simulink model "AuxClimaCtlMgr"  (R2022b)
settings: Solver=FixedStepDiscrete  StartTime=0.0  StopTime=10.0  FixedStep=0.2  RelTol=1e-3
(part 1 of 2)
settings Model: Creator=blue.ge  LastModifiedBy=blue.ge
settings DataIOCC: SaveFormat=StructureWithTime
settings OptimizationCC: DefaultParameterBehavior=Inlined  EnhancedBackFolding=on  PassReuseOutputArgsAs=Individual arguments  ZeroExternalMemoryAtStartup=off  ZeroInternalMemoryAtStartup=off  OptimizeBlockOrder=speed  DifferentSizesBufferReuse=on  OptimizationCustomize=off  EfficientTunableParamExpr=on
settings DebuggingCC: ParameterTunabilityLossMsg=error
settings ModelReferenceCC: ModelReferenceNumInstancesAllowed=Single
settings SFSimCC: MATLABDynamicMemAlloc=off
settings RTWCC: Description=AUTOSAR  SystemTargetFile=autosar.tlc  GenCodeOnly=on  TemplateMakefile=ert_default_tmf
settings CodeAppCC: ForceParamTrailComments=on  IgnoreCustomStorageClasses=off  OperatorAnnotations=on  SimulinkDataObjDesc=on  SFDataObjDesc=on  InsertBlockDesc=on
settings STFCustomTargetCC: TargetLangStandard=C99 (ISO)  MultiwordLength=256  ParMdlRefBuildCompliant=on  CompOptLevelCompliant=on  IncludeMdlTerminateFcn=off  CombineOutputUpdateFcns=on  SuppressErrorStatus=on  ERTCustomFileBanners=on  SupportNonFinite=off  SupportComplex=off  SupportContinuousTime=off  SupportNonInlinedSFcns=off  RemoveResetFunc=on  MATLABClassNameForMDSCustomization=Simulink.SoftwareTarget.ERTCustomization  AutosarCompliant=on  UseToolchainInfoCompliant=on  LookupTableObjectStructAxisOrder=2,1,3,4,...  LUTObjectStructOrderEvenSpacing=Size,Table,Breakpoints  SystemTargetFile=autosar.tlc
settings ERTTargetCC: TargetLangStandard=C99 (ISO)  ConcurrentExecutionCompliant=off  IncludeMdlTerminateFcn=off  SuppressErrorStatus=on  SupportNonFinite=off  SupportComplex=off  ModelStepFunctionPrototypeControlCompliant=off  CPPClassGenCompliant=off  AutosarCompliant=on  LookupTableObjectStructAxisOrder=2,1,3,4,...  LUTObjectStructOrderEvenSpacing=Size,Table,Breakpoints  InlinedParameterPlacement=NonHierarchical  ERTCustomFileTemplate=example_file_process.tlc

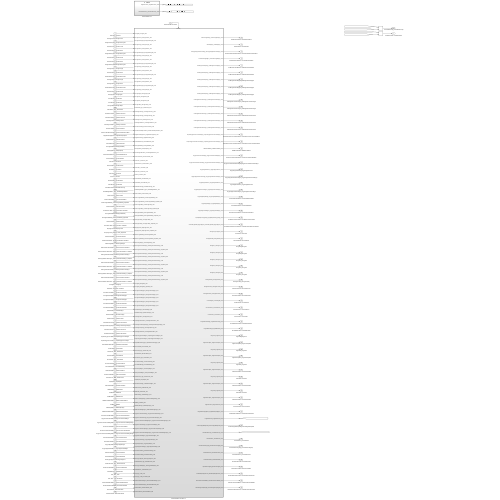
[thumbnail: Simulink's stored preview of the top-level diagram]
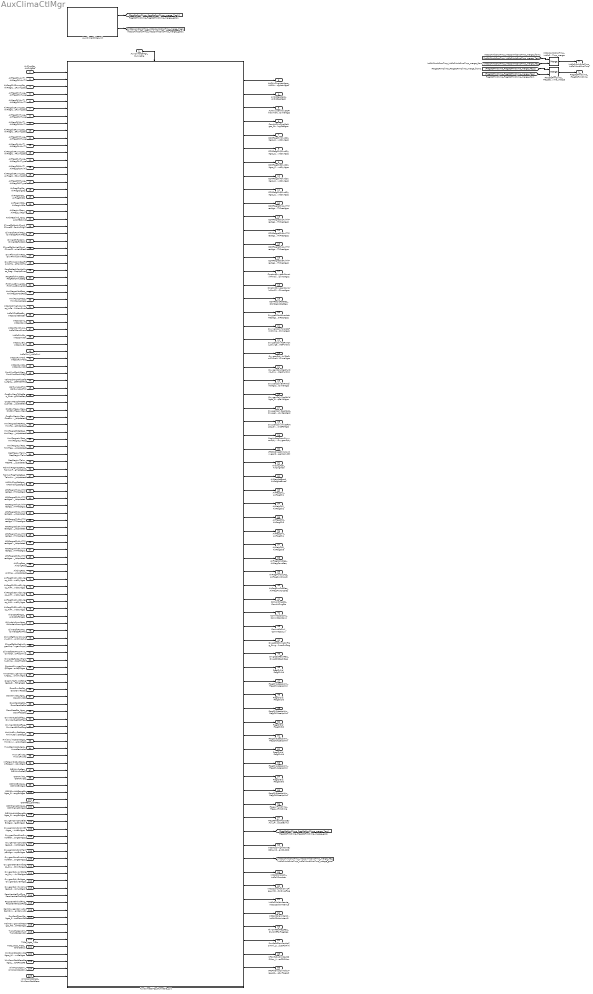
== system AuxClimaCtlMgr ==
  AuxClimaCtlMgr_Runnable : Inport  SampleTime=0.2  OutputFunctionCall=on
  AirClngSts_AirClngSts : Inport  Port=2  OutDataTypeStr=Enum: AirIonSts  PortDimensions=1
  AirFragCh1AvlTi_AirFragCh1AvlTi : Inport  Port=3  OutMin=0  OutMax=360  OutDataTypeStr=AfuTime  PortDimensions=1
  AirFragCh1RunngSts_AirFragCh1RunngSts : Inport  Port=4  OutDataTypeStr=Enum: OnOff1  PortDimensions=1
  AirFragCh1Type_AirFragCh1Type : Inport  Port=5  OutDataTypeStr=UInt8  PortDimensions=1
  AirFragCh2AvlTi_AirFragCh2AvlTi : Inport  Port=6  OutMin=0  OutMax=360  OutDataTypeStr=AfuTime  PortDimensions=1
  AirFragCh2RunngSts_AirFragCh2RunngSts : Inport  Port=7  OutDataTypeStr=Enum: OnOff1  PortDimensions=1
  AirFragCh2Type_AirFragCh2Type : Inport  Port=8  OutDataTypeStr=UInt8  PortDimensions=1
  AirFragCh3AvlTi_AirFragCh3AvlTi : Inport  Port=9  OutMin=0  OutMax=360  OutDataTypeStr=AfuTime  PortDimensions=1
  AirFragCh3RunngSts_AirFragCh3RunngSts : Inport  Port=10  OutDataTypeStr=Enum: OnOff1  PortDimensions=1
  AirFragCh3Type_AirFragCh3Type : Inport  Port=11  OutDataTypeStr=UInt8  PortDimensions=1
  AirFragCh4AvlTi_AirFragCh4AvlTi : Inport  Port=12  OutMin=0  OutMax=360  OutDataTypeStr=AfuTime  PortDimensions=1
  AirFragCh4RunngSts_AirFragCh4RunngSts : Inport  Port=13  OutDataTypeStr=Enum: OnOff1  PortDimensions=1
  AirFragCh4Type_AirFragCh4Type : Inport  Port=14  OutDataTypeStr=UInt8  PortDimensions=1
  AirFragCh5AvlTi_AirFragCh5AvlTi : Inport  Port=15  OutMin=0  OutMax=360  OutDataTypeStr=AfuTime  PortDimensions=1
  AirFragCh5RunngSts_AirFragCh5RunngSts : Inport  Port=16  OutDataTypeStr=Enum: OnOff1  PortDimensions=1
  AirFragCh5Type_AirFragCh5Type : Inport  Port=17  OutDataTypeStr=UInt8  PortDimensions=1
  AirFragChgSts_AirFragChgSts : Inport  Port=18  OutDataTypeStr=Enum: ChagSts  PortDimensions=1
  AirFragErrSts_AirFragErrSts : Inport  Port=19  OutDataTypeStr=Boolean  PortDimensions=1
  AirFragInitSts_AirFragInitSts : Inport  Port=20  OutDataTypeStr=Enum: InitSts  PortDimensions=1
  AirFragLvlRspn_AirFragLvlRspn : Inport  Port=21  OutDataTypeStr=Enum: RatUse  PortDimensions=1
  AmbTEstimd_Ngcc_AmbTEstimd : Inport  Port=22  OutDataTypeStr=Bus: AmbTEstimd  PortDimensions=1  BusOutputAsStruct=on
  ClimaCfgForAirClng1_ClimaCfgForAirClng1 : Inport  Port=23  OutDataTypeStr=Boolean  PortDimensions=1
  ClimaCfgForAirFrag_ClimaCfgForAirFrag : Inport  Port=24  OutDataTypeStr=Boolean  PortDimensions=1
  ClimaCfgForCds1_ClimaCfgForCds1 : Inport  Port=25  OutDataTypeStr=Boolean  PortDimensions=1
  ClimaCfgForVehElec1_ClimaCfgForVehElec1 : Inport  Port=26  OutDataTypeStr=Boolean  PortDimensions=1
  DrvrPfmncAlrmReq_DrvrPfmncAlrmReq : Inport  Port=27  OutDataTypeStr=Enum: DrvrPfmncWarnReq  PortDimensions=1
  DrvrPfmncAlrmReqFromDHU_DrvrPfmncAlrmReqFromDHU : Inport  Port=28  OutDataTypeStr=Enum: DrvrPfmncWarnReq  PortDimensions=1
  FragRefreshPopUpSucs_FragRefreshPopUpSucs : Inport  Port=29  OutDataTypeStr=Boolean  PortDimensions=1
  FragRefrshAutSetg_FragRefrshAutSetg : Inport  Port=30  OutDataTypeStr=Enum: OnOff1  PortDimensions=1
  FrntHvacBlowerSts_FrntHvacBlowerSts : Inport  Port=31  OutDataTypeStr=Enum: HvacFanSts  PortDimensions=1
  HmiFragraModReq_HmiFragraModReq : Inport  Port=32  OutDataTypeStr=Enum: HmiFragraModReq  PortDimensions=1
  HmiPopUpResp_HmiPopUpResp : Inport  Port=33  OutDataTypeStr=Enum: BookChargeSetResponse  PortDimensions=1
  InCarCo2HighWarnSucs_InCarCo2HighWarnSucs : Inport  Port=34  OutDataTypeStr=Boolean  PortDimensions=1
  IntCo2CircElecErr_IntCo2CircElecErr : Inport  Port=35  OutDataTypeStr=Enum: PmSnsrErrSts  PortDimensions=1
  IntCo2Conc_IntCo2Conc : Inport  Port=36  OutDataTypeStr=Co2Conc  PortDimensions=1
  IntCo2fromExsrc_IntCo2fromExsrc : Inport  Port=37  OutDataTypeStr=Enum: OnOff1  PortDimensions=1
  IntCo2HvErr_IntCo2HvErr : Inport  Port=38  OutDataTypeStr=Enum: Err1  PortDimensions=1
  IntCo2LvErr_IntCo2LvErr : Inport  Port=39  OutDataTypeStr=Enum: Err1  PortDimensions=1
  IntCo2Lvl_IntCo2Lvl : Inport  Port=40  OutDataTypeStr=Enum: Co2Lvl  PortDimensions=1
  IntCo2RunMod_IntCo2RunMod : Inport  Port=41  OutDataTypeStr=Enum: CdsRunngMod  PortDimensions=1
  IntCo2RunSts_IntCo2RunSts : Inport  Port=42  OutDataTypeStr=Enum: PmSnsrSts  PortDimensions=1
  PwrAllwdForAirFrag_PwrAllwdForAirFrag : Inport  Port=43  OutDataTypeStr=Pwr33  PortDimensions=1
  VehModMngtGlbSafe1_Ngcc_VehModMngtGlbSafe1 : Inport  Port=44  OutDataTypeStr=Bus: VehModMngtGlbSafe1  PortDimensions=1  BusOutputAsStruct=on
  VehSunLoadTot_VehSunLoadTot : Inport  Port=45  OutDataTypeStr=SunLoad30  PortDimensions=1
  CnctSrvFragChRatReq_CnctSrvFragChRatReq : Inport  Port=46  OutDataTypeStr=Bus: HmiFragraTypRatReq  PortDimensions=1  BusOutputAsStruct=on
  CnctSrvFragChRatReq_CnctSrvFragChRatReq_IsUpdated : Inport  Port=47  OutDataTypeStr=boolean  PortDimensions=1
  CnctSrvFragLvlReq_CnctSrvFragLvlReq : Inport  Port=48  OutDataTypeStr=Enum: HmiFragraLvlReq  PortDimensions=1
  CnctSrvFragLvlReq_CnctSrvFragLvlReq_IsUpdated : Inport  Port=49  OutDataTypeStr=boolean  PortDimensions=1
  HmiFragraChRatReq_HmiFragraChRatReq : Inport  Port=50  OutDataTypeStr=Bus: HmiFragraTypRatReq  PortDimensions=1  BusOutputAsStruct=on
  HmiFragraChRatReq_HmiFragraChRatReq_IsUpdated : Inport  Port=51  OutDataTypeStr=boolean  PortDimensions=1
  HmiFragraLvlReq_HmiFragraLvlReq : Inport  Port=52  OutDataTypeStr=Enum: HmiFragraLvlReq  PortDimensions=1
  HmiFragraLvlReq_HmiFragraLvlReq_IsUpdated : Inport  Port=53  OutDataTypeStr=boolean  PortDimensions=1
  ReqFragLvlTelm_ReqFragLvlTelm : Inport  Port=54  OutDataTypeStr=Enum: ReqFragLvlTelm  PortDimensions=1
  ReqFragLvlTelm_ReqFragLvlTelm_IsUpdated : Inport  Port=55  OutDataTypeStr=boolean  PortDimensions=1
  TelmAirFragTasteReq_TelmAirFragTasteReq : Inport  Port=56  OutDataTypeStr=Enum: TelmAirFragChReq  PortDimensions=1
  TelmAirFragTasteReq_TelmAirFragTasteReq_IsUpdated : Inport  Port=57  OutDataTypeStr=boolean  PortDimensions=1
  AACAirClngStsNgcc_AACAirClngStsNgcc : Inport  Port=58  OutDataTypeStr=Enum: AACAirClngSts  PortDimensions=1
  AFUFragraCh1AvlTiPrecNgcc_AFUFragraCh1AvlTiPrecNgcc : Inport  Port=59  OutMin=0  OutMax=100  OutDataTypeStr=Perc9  PortDimensions=1
  AFUFragraCh1AvlTiPrecNgcc_AFUFragraCh1AvlTiPrecNgcc_IsUpdated : Inport  Port=60  OutDataTypeStr=boolean  PortDimensions=1
  AFUFragraCh2AvlTiPrecNgcc_AFUFragraCh2AvlTiPrecNgcc : Inport  Port=61  OutMin=0  OutMax=100  OutDataTypeStr=Perc9  PortDimensions=1
  AFUFragraCh2AvlTiPrecNgcc_AFUFragraCh2AvlTiPrecNgcc_IsUpdated : Inport  Port=62  OutDataTypeStr=boolean  PortDimensions=1
  AFUFragraCh3AvlTiPrecNgcc_AFUFragraCh3AvlTiPrecNgcc : Inport  Port=63  OutMin=0  OutMax=100  OutDataTypeStr=Perc9  PortDimensions=1
  AFUFragraCh3AvlTiPrecNgcc_AFUFragraCh3AvlTiPrecNgcc_IsUpdated : Inport  Port=64  OutDataTypeStr=boolean  PortDimensions=1
  AFUFragraCh4AvlTiPrecNgcc_AFUFragraCh4AvlTiPrecNgcc : Inport  Port=65  OutMin=0  OutMax=100  OutDataTypeStr=Perc9  PortDimensions=1
  AFUFragraCh4AvlTiPrecNgcc_AFUFragraCh4AvlTiPrecNgcc_IsUpdated : Inport  Port=66  OutDataTypeStr=boolean  PortDimensions=1
  AFUFragraCh5AvlTiPrecNgcc_AFUFragraCh5AvlTiPrecNgcc : Inport  Port=67  OutMin=0  OutMax=100  OutDataTypeStr=Perc9  PortDimensions=1
  AFUFragraCh5AvlTiPrecNgcc_AFUFragraCh5AvlTiPrecNgcc_IsUpdated : Inport  Port=68  OutDataTypeStr=boolean  PortDimensions=1
  AirClngReq_AirClngReq : Inport  Port=69  OutDataTypeStr=Enum: OnOffNoReq  PortDimensions=1
  AirClngReq_AirClngReq_IsUpdated : Inport  Port=70  OutDataTypeStr=boolean  PortDimensions=1
  AirFragCh1OvrdDyNgcc_AirFragCh1OvrdDyNgcc : Inport  Port=71  OutDataTypeStr=Bus: DateTi30  PortDimensions=1  BusOutputAsStruct=on
  AirFragCh2OvrdDyNgcc_AirFragCh2OvrdDyNgcc : Inport  Port=72  OutDataTypeStr=Bus: DateTi30  PortDimensions=1  BusOutputAsStruct=on
  AirFragCh3OvrdDyNgcc_AirFragCh3OvrdDyNgcc : Inport  Port=73  OutDataTypeStr=Bus: DateTi30  PortDimensions=1  BusOutputAsStruct=on
  AirFragCh4OvrdDyNgcc_AirFragCh4OvrdDyNgcc : Inport  Port=74  OutDataTypeStr=Bus: DateTi30  PortDimensions=1  BusOutputAsStruct=on
  AirFragCh5OvrdDyNgcc_AirFragCh5OvrdDyNgcc : Inport  Port=75  OutDataTypeStr=Bus: DateTi30  PortDimensions=1  BusOutputAsStruct=on
  AirSrcStsFbNgcc_AirSrcStsFbNgcc : Inport  Port=76  OutMin=0  OutMax=3  OutDataTypeStr=UInt2  PortDimensions=1
  AltitudeInfromNgcc_AltitudeInfromNgcc : Inport  Port=77  OutDataTypeStr=UInt15  PortDimensions=1
  ClimaCfgForAAC_ClimaCfgForAAC : Inport  Port=78  OutDataTypeStr=Enum: CarCfgForAirClng  PortDimensions=1
  ClimaCfgForAirClnrAdv1_ClimaCfgForAirClnrAdv1 : Inport  Port=79  OutDataTypeStr=Boolean  PortDimensions=1
  ClimaCfgForCabinOxgenSupply_ClimaCfgForCabinOxgenSupply : Inport  Port=80  OutDataTypeStr=Enum: CabinOxgenSupply  PortDimensions=1
  ClimaCfgForPrpsnTyp_ClimaCfgForPrpsnTyp : Inport  Port=81  OutDataTypeStr=Enum: CarCfgForPrpsnTypGly  PortDimensions=1
  ClimaCfgForRoofHatch_ClimaCfgForRoofHatch : Inport  Port=82  OutDataTypeStr=Enum: CarCfgForRoofHatch  PortDimensions=1
  CmptmtOxygenConcQFNgcc_CmptmtOxygenConcQFNgcc : Inport  Port=83  OutDataTypeStr=Enum: Qf1  PortDimensions=1
  CmptmtOxygenConcVluNgcc_CmptmtOxygenConcVluNgcc : Inport  Port=84  OutMin=0  OutMax=100  OutDataTypeStr=Perc9  PortDimensions=1
  DispHvBattLvlOfChrgNgcc_DispHvBattLvlOfChrgNgcc : Inport  Port=85  OutMin=0  OutMax=102.30000000000001  OutDataTypeStr=EcoDrvInfo  PortDimensions=1
  DoorDrvrReSts_DoorDrvrReSts : Inport  Port=86  OutDataTypeStr=Enum: DoorSts2  PortDimensions=1
  DoorDrvrSts_Ngcc_DoorDrvrSts : Inport  Port=87  OutDataTypeStr=Enum: DoorSts2  PortDimensions=1
  DoorPassReSts_DoorPassReSts : Inport  Port=88  OutDataTypeStr=Enum: DoorSts2  PortDimensions=1
  DoorPassSts_Ngcc_DoorPassSts : Inport  Port=89  OutDataTypeStr=Enum: DoorSts2  PortDimensions=1
  DrvrVentLeClsdFlag_DrvrVentLeClsdFlag : Inport  Port=90  OutDataTypeStr=Boolean  PortDimensions=1
  DrvrVentRiClsdFlag_DrvrVentRiClsdFlag : Inport  Port=91  OutDataTypeStr=Boolean  PortDimensions=1
  HmiAutOxySetNgcc_HmiAutOxySetNgcc : Inport  Port=92  OutDataTypeStr=Enum: OnOff1  PortDimensions=1
  HmiOxyinCarSwtNgcc_HmiOxyinCarSwtNgcc : Inport  Port=93  OutDataTypeStr=Enum: NoReqOnOff  PortDimensions=1
  HvacRecircAct_Ngcc_HvacRecircAct : Inport  Port=94  OutDataTypeStr=Perc4  PortDimensions=1
  HvSysRlySts_HvSysRlySts : Inport  Port=95  OutDataTypeStr=Bus: HvSysRlySts  PortDimensions=1  BusOutputAsStruct=on
  InflateAirSrcSwtNgcc_InflateAirSrcSwtNgcc : Inport  Port=96  OutDataTypeStr=Enum: OnOffNoReq  PortDimensions=1
  OGMAirSrcReq_OGMAirSrcReq : Inport  Port=97  OutDataTypeStr=Enum: OnOffNoReq  PortDimensions=1
  OGMAvlSts_OGMAvlSts : Inport  Port=98  OutDataTypeStr=Enum: OxygenSplyAvlSts  PortDimensions=1
  OGMIntErrNgcc_OGMIntErrNgcc : Inport  Port=99  OutDataTypeStr=Enum: Err1  PortDimensions=1
  OGMOvrVoltRangErrNgcc_OGMOvrVoltRangErrNgcc : Inport  Port=100  OutDataTypeStr=Enum: Err1  PortDimensions=1
  OGMReq_OGMReq : Inport  Port=101  OutDataTypeStr=Enum: OxygenSplyReq  PortDimensions=1
  OGMTempErrNgcc_OGMTempErrNgcc : Inport  Port=102  OutDataTypeStr=Enum: TempErr  PortDimensions=1
  OGMUndVoltRangErrNgcc_OGMUndVoltRangErrNgcc : Inport  Port=103  OutDataTypeStr=Enum: Err1  PortDimensions=1
  OxygenConcSnsrElecErrNgcc_OxygenConcSnsrElecErrNgcc : Inport  Port=104  OutDataTypeStr=Enum: OxygenConcSnsrElecErr  PortDimensions=1
  OxygenConcSnsrIntErrNgcc_OxygenConcSnsrIntErrNgcc : Inport  Port=105  OutDataTypeStr=Enum: Err1  PortDimensions=1
  OxygenConcSnsrOvrVoltRangErrNgcc_OxygenConcSnsrOvrVoltRangErrNgcc : Inport  Port=106  OutDataTypeStr=Enum: Err1  PortDimensions=1
  OxygenConcSnsrStsNgcc_OxygenConcSnsrStsNgcc : Inport  Port=107  OutDataTypeStr=Enum: PmSnsrSts  PortDimensions=1
  OxygenConcSnsrTempErrNgcc_OxygenConcSnsrTempErrNgcc : Inport  Port=108  OutDataTypeStr=Enum: TempErr  PortDimensions=1
  OxygenConcSnsrUndVoltRangErrNgcc_OxygenConcSnsrUndVoltRangErrNgcc : Inport  Port=109  OutDataTypeStr=Enum: Err1  PortDimensions=1
  OxygenGenrErrInfoNgcc_OxygenGenrErrInfoNgcc : Inport  Port=110  OutDataTypeStr=Enum: OxygengenrErrInfo  PortDimensions=1
  OxygenSplyAvlStsNgcc_OxygenSplyAvlStsNgcc : Inport  Port=111  OutDataTypeStr=Enum: OxygenSplyAvlSts  PortDimensions=1
  OxygenSplyErrNgcc_OxygenSplyErrNgcc : Inport  Port=112  OutDataTypeStr=Enum: Err1  PortDimensions=1
  OxygenSplyPwrAllwdNgcc_OxygenSplyPwrAllwdNgcc : Inport  Port=113  OutMin=0  OutMax=2047  OutDataTypeStr=Pwr30  PortDimensions=1
  PassVentLeClsdFlag_PassVentLeClsdFlag : Inport  Port=114  OutDataTypeStr=Boolean  PortDimensions=1
  PassVentRiClsdFlag_PassVentRiClsdFlag : Inport  Port=115  OutDataTypeStr=Boolean  PortDimensions=1
  RemOxygenSplyActv_RemOxygenSplyActv : Inport  Port=116  OutDataTypeStr=Enum: OnOff1  PortDimensions=1
  SunRoofPosnSts_Ngcc_SunRoofPosnSts : Inport  Port=117  OutDataTypeStr=Enum: WinAndRoofAndCurtPosnTyp  PortDimensions=1
  TelmOxygenValReqNgcc_TelmOxygenValReqNgcc : Inport  Port=118  OutDataTypeStr=Enum: OnOffNoReq  PortDimensions=1
  TiAndDateIndcn_TiAndDateIndcn : Inport  Port=119  OutDataTypeStr=Bus: DateTi30  PortDimensions=1  BusOutputAsStruct=on
  TrSts_Ngcc_TrSts : Inport  Port=120  OutDataTypeStr=Enum: DoorSts2  PortDimensions=1
  TrSts_Ngcc_TrSts_ErrorStatus : Inport  Port=121  OutDataTypeStr=uint8  PortDimensions=1
  WinPosnStsAtDrvrReNgcc_WinPosnStsAtDrvrReNgcc : Inport  Port=122  OutDataTypeStr=Enum: WinAndRoofAndCurtPosnTyp  PortDimensions=1
  WinPosnStsAtPassReNgcc_WinPosnStsAtPassRe : Inport  Port=123  OutDataTypeStr=Enum: WinAndRoofAndCurtPosnTyp  PortDimensions=1
  WinPosnStsDrv_WinPosnStsAtDrv : Inport  Port=124  OutDataTypeStr=Enum: WinAndRoofAndCurtPosnTyp  PortDimensions=1
  WinPosnStsPass_WinPosnStsAtPass : Inport  Port=125  OutDataTypeStr=Enum: WinAndRoofAndCurtPosnTyp  PortDimensions=1
  AuxClimaCtlMgr_Init : Subsystem
  AuxClimaCtlMgr_Runnable_sys : Subsystem
  FragStsFrmClima_FragStsFrmClima_merge : Merge
  IntCo2AmbSnsrTmp_IntCo2AmbSnsrTmp_merge : Merge
  FragStsFrmClima_FragStsFrmClima : Outport  OutDataTypeStr=Enum: AirFragSts  PortDimensions=1
  IntCo2AmbSnsrTmp_IntCo2AmbSnsrTmp : Outport  Port=2  OutDataTypeStr=Bus: AmbTEstimd  PortDimensions=1  BusOutputAsStruct=on
  AACAirClngActnNgcc_AACAirClngActnNgcc : Outport  Port=3  OutDataTypeStr=Enum: OnOff1  PortDimensions=1
  AirSrcReqNgcc_AirSrcReqNgcc : Outport  Port=4  OutDataTypeStr=Enum: OnOffNoReq  PortDimensions=1
  AutOxygenSplyStsFrmClimaNgcc_AutOxygenSplyStsFrmClimaNgcc : Outport  Port=5  OutDataTypeStr=Enum: OnOff1  PortDimensions=1
  CcmAACAirClngStsNgcc_CcmAACAirClngStsNgcc : Outport  Port=6  OutDataTypeStr=Enum: AACAirClngSts  PortDimensions=1
  CCMFragCh1OvrdDyNgcc_CCMFragCh1OvrdDyNgcc : Outport  Port=7  OutDataTypeStr=Enum: Warn2  PortDimensions=1
  CCMFragCh2OvrdDyNgcc_CCMFragCh2OvrdDyNgcc : Outport  Port=8  OutDataTypeStr=Enum: Warn2  PortDimensions=1
  CCMFragCh3OvrdDyNgcc_CCMFragCh3OvrdDyNgcc : Outport  Port=9  OutDataTypeStr=Enum: Warn2  PortDimensions=1
  CCMFragCh4OvrdDyNgcc_CCMFragCh4OvrdDyNgcc : Outport  Port=10  OutDataTypeStr=Enum: Warn2  PortDimensions=1
  CCMFragCh5OvrdDyNgcc_CCMFragCh5OvrdDyNgcc : Outport  Port=11  OutDataTypeStr=Enum: Warn2  PortDimensions=1
  CCMFragraCh1AvlTiPrecNgcc_CCMFragraCh1AvlTiPrecNgcc : Outport  Port=12  OutMin=0  OutMax=100  OutDataTypeStr=Perc9  PortDimensions=1
  CCMFragraCh2AvlTiPrecNgcc_CCMFragraCh2AvlTiPrecNgcc : Outport  Port=13  OutMin=0  OutMax=100  OutDataTypeStr=Perc9  PortDimensions=1
  CCMFragraCh3AvlTiPrecNgcc_CCMFragraCh3AvlTiPrecNgcc : Outport  Port=14  OutMin=0  OutMax=100  OutDataTypeStr=Perc9  PortDimensions=1
  CCMFragraCh4AvlTiPrecNgcc_CCMFragraCh4AvlTiPrecNgcc : Outport  Port=15  OutMin=0  OutMax=100  OutDataTypeStr=Perc9  PortDimensions=1
  CCMFragraCh5AvlTiPrecNgcc_CCMFragraCh5AvlTiPrecNgcc : Outport  Port=16  OutMin=0  OutMax=100  OutDataTypeStr=Perc9  PortDimensions=1
  CmptmtOxygenConcLvlFrmClimaNgcc_CmptmtOxygenConcLvlFrmClimaNgcc : Outport  Port=17  OutDataTypeStr=Enum: UnknowLvl  PortDimensions=1
  CmptmtOxygenConcVluFrmClimaNgcc_CmptmtOxygenConcVluFrmClimaNgcc : Outport  Port=18  OutMin=0  OutMax=100  OutDataTypeStr=Perc9  PortDimensions=1
  OSMRecircRatReq_OSMRecircRatReq : Outport  Port=19  OutMin=0  OutMax=2047  OutDataTypeStr=Pwr30  PortDimensions=1
  OxygenConcSnsrActnReqNgcc_OxygenConcSnsrActnReqNgcc : Outport  Port=20  OutDataTypeStr=Enum: OnOff1  PortDimensions=1
  OxygenConcSnsrStsFrmClimaNgcc_OxygenConcSnsrStsFrmClimaNgcc : Outport  Port=21  OutDataTypeStr=Enum: PmSnsrSts  PortDimensions=1
  OxygenSplyActPwrCns_OxygenSplyActPwrCns : Outport  Port=22  OutMin=0  OutMax=2047  OutDataTypeStr=Pwr30  PortDimensions=1
  OxygenSplyAvlStsFrmClimaNgcc_OxygenSplyAvlStsFrmClimaNgcc : Outport  Port=23  OutDataTypeStr=Enum: OxygenSplyAvlSts  PortDimensions=1
  OxygenSplyDesPwrCns_OxygenSplyDesPwrCns : Outport  Port=24  OutMin=0  OutMax=2047  OutDataTypeStr=Pwr30  PortDimensions=1
  OxygenSplyErrFrmClimaNgcc_OxygenSplyErrFrmClimaNgcc : Outport  Port=25  OutDataTypeStr=Enum: Err1  PortDimensions=1
  OxygenSplyReqFaildNgcc_OxygenSplyReqFaildNgcc : Outport  Port=26  OutMin=0  OutMax=3  OutDataTypeStr=UInt2  PortDimensions=1
  OxygenSplyReqNgcc_OxygenSplyReqNgcc : Outport  Port=27  OutDataTypeStr=Enum: OxygenSplyReq  PortDimensions=1
  OxygenSplyVoiceFbNgcc_OxygenSplyVoiceFbNgcc : Outport  Port=28  OutDataTypeStr=Enum: OxygenSplyVoiceFb  PortDimensions=1
  PopUpReqFromOxygenSply_PopUpReqFromOxygenSply : Outport  Port=29  OutDataTypeStr=Enum: OxygenSplyPopUpReq  PortDimensions=1
  VFCPrkDrvgClimabyOxygenSplyCtrl_VFCPrkDrvgClimabyOxygenSplyCtrl : Outport  Port=30  OutDataTypeStr=Boolean  PortDimensions=1
  AirClngActn_AirClngActn : Outport  Port=31  OutDataTypeStr=Enum: OnOff1  PortDimensions=1
  AirFragActEnad_AirFragActEnad : Outport  Port=32  OutDataTypeStr=Enum: OnOff1  PortDimensions=1
  AirFragCh1_AirFragCh1 : Outport  Port=33  OutDataTypeStr=Enum: OnOff1  PortDimensions=1
  AirFragCh2_AirFragCh2 : Outport  Port=34  OutDataTypeStr=Enum: OnOff1  PortDimensions=1
  AirFragCh3_AirFragCh3 : Outport  Port=35  OutDataTypeStr=Enum: OnOff1  PortDimensions=1
  AirFragCh4_AirFragCh4 : Outport  Port=36  OutDataTypeStr=Enum: OnOff1  PortDimensions=1
  AirFragCh5_AirFragCh5 : Outport  Port=37  OutDataTypeStr=Enum: OnOff1  PortDimensions=1
  AirFragConcReq_AirFragConcReq : Outport  Port=38  OutDataTypeStr=Enum: ReqFragLvlTelm  PortDimensions=1
  AirFragPwrCnsAct_AirFragPwrCnsAct : Outport  Port=39  OutDataTypeStr=Pwr33  PortDimensions=1
  AirFragPwrCnsDes_AirFragPwrCnsDes : Outport  Port=40  OutDataTypeStr=Pwr33  PortDimensions=1
  CcmAirClngSts_CcmAirClngSts : Outport  Port=41  OutDataTypeStr=Enum: AirIonSts  PortDimensions=1
  CcmIntCo2Conc_CcmIntCo2Conc : Outport  Port=42  OutDataTypeStr=Co2Conc  PortDimensions=1
  CcmIntCo2Lvl_CcmIntCo2Lvl : Outport  Port=43  OutDataTypeStr=Enum: Co2Lvl  PortDimensions=1
  DrvgCDSClimaOnFlag_DrvgCDSClimaOnFlag : Outport  Port=44  OutDataTypeStr=Boolean  PortDimensions=1
  DrvgCDSRecirReq_DrvgCDSRecirReq : Outport  Port=45  OutDataTypeStr=Perc30  PortDimensions=1
  FragCh1Id_FragCh1Id : Outport  Port=46  OutDataTypeStr=UInt8  PortDimensions=1
  FragCh1UseUpWrn_FragCh1UseUpWrn : Outport  Port=47  OutDataTypeStr=Enum: Warn2  PortDimensions=1
  FragCh2Id_FragCh2Id : Outport  Port=48  OutDataTypeStr=UInt8  PortDimensions=1
  FragCh2UseUpWrn_FragCh2UseUpWrn : Outport  Port=49  OutDataTypeStr=Enum: Warn2  PortDimensions=1
  FragCh3Id_FragCh3Id : Outport  Port=50  OutDataTypeStr=UInt8  PortDimensions=1
  FragCh3UseUpWrn_FragCh3UseUpWrn : Outport  Port=51  OutDataTypeStr=Enum: Warn2  PortDimensions=1
  FragCh4Id_FragCh4Id : Outport  Port=52  OutDataTypeStr=UInt8  PortDimensions=1
  FragCh4UseUpWrn_FragCh4UseUpWrn : Outport  Port=53  OutDataTypeStr=Enum: Warn2  PortDimensions=1
  FragCh5Id_FragCh5Id : Outport  Port=54  OutDataTypeStr=UInt8  PortDimensions=1
  FragCh5UseUpWrn_FragCh5UseUpWrn : Outport  Port=55  OutDataTypeStr=Enum: Warn2  PortDimensions=1
  FragLvlFrmClima_FragLvlFrmClima : Outport  Port=56  OutDataTypeStr=Enum: RatUse  PortDimensions=1
  FragRefreshPopUpByAux_FragRefreshPopUpByAux : Outport  Port=57  OutDataTypeStr=Enum: OnOff1  PortDimensions=1
  InCarCo2HighWarnByCDS_InCarCo2HighWarnByCDS : Outport  Port=58  OutDataTypeStr=Enum: Co2Lvl  PortDimensions=1
  IntCo2SnsrActv_IntCo2SnsrActv : Outport  Port=59  OutDataTypeStr=Enum: ReqSts1  PortDimensions=1
  IntCo2SnsrActvDrvgFlag_IntCo2SnsrActvDrvgFlag : Outport  Port=60  OutDataTypeStr=Boolean  PortDimensions=1
  IntCo2SolarIntenLe_IntCo2SolarIntenLe : Outport  Port=61  OutDataTypeStr=Sunload2D  PortDimensions=1
  IntCo2SolarIntenRi_IntCo2SolarIntenRi : Outport  Port=62  OutDataTypeStr=Sunload2D  PortDimensions=1
  RlyCmftForFragReq_RlyCmftForFragReq : Outport  Port=63  OutDataTypeStr=Enum: OnOff1  PortDimensions=1
  SunRoofOpnPopUpForHmi_SunRoofOpnPopUpForHmi : Outport  Port=64  OutDataTypeStr=Enum: ReqSts1  PortDimensions=1
  VFCInfoPushInCarCDSMon_VFCInfoPushInCarCDSMon : Outport  Port=65  OutDataTypeStr=Boolean  PortDimensions=1